simulink model "SdbTxSigProc"  (R2022b) (continued)
== system SdbTxSigProc/SdbTxSigProc_Runnable_sys == (continued)
  HeatrAirTReq_HeatrAirTReq_write : Outport  Port=155
      settings: EnsureOutportIsVirtual=on
  HvacAirMFlowEstimd_HvacAirMFlowEstimd_write : Outport  Port=156
      settings: EnsureOutportIsVirtual=on
  HvacAirMFlowReEstimd_HvacAirMFlowReEstimd_write : Outport  Port=157
      settings: EnsureOutportIsVirtual=on
  HvacAirMFlowVent_HvacAirMFlowVent_write : Outport  Port=158
      settings: EnsureOutportIsVirtual=on
  HvacAirTForRowFirstAtFlrLe_HvacAirTForRowFirstAtFlrLe_write : Outport  Port=159
      settings: EnsureOutportIsVirtual=on
  HvacAirTForRowFirstAtFlrRi_HvacAirTForRowFirstAtFlrRi_write : Outport  Port=160
      settings: EnsureOutportIsVirtual=on
  HvacAirTForRowFirstAtVentLe_HvacAirTForRowFirstAtVentLe_write : Outport  Port=161
      settings: EnsureOutportIsVirtual=on
  HvacAirTForRowFirstAtVentRi_HvacAirTForRowFirstAtVentRi_write : Outport  Port=162
      settings: EnsureOutportIsVirtual=on
  HvacAirTForRowSecAtFlrLe_HvacAirTForRowSecAtFlrLe_write : Outport  Port=163
      settings: EnsureOutportIsVirtual=on
  HvacAirTForRowSecAtFlrRi_HvacAirTForRowSecAtFlrRi_write : Outport  Port=164
      settings: EnsureOutportIsVirtual=on
  HvacAirTForRowSecAtVentLe_HvacAirTForRowSecAtVentLe_write : Outport  Port=165
      settings: EnsureOutportIsVirtual=on
  HvacAirTForRowSecAtVentRi_HvacAirTForRowSecAtVentRi_write : Outport  Port=166
      settings: EnsureOutportIsVirtual=on
  HvacCondsorTsp_HvacCondsorTsp_write : Outport  Port=167
      settings: EnsureOutportIsVirtual=on
  HvacCoolgEnaFrnt_HvacCoolgEnaFrnt_write : Outport  Port=168
      settings: EnsureOutportIsVirtual=on
  HvacCoolgEnaRe_HvacCoolgEnaRe_write : Outport  Port=169
      settings: EnsureOutportIsVirtual=on
  HvacEvaprTSpFrnt_HvacEvaprTSpFrnt_write : Outport  Port=170
      settings: EnsureOutportIsVirtual=on
  HvacHeatElmAirMFlowEstimd_HvacHeatElmAirMFlowEstimd_write : Outport  Port=171
      settings: EnsureOutportIsVirtual=on
  HvacHeatgReq_HvacHeatgReq_write : Outport  Port=172
      settings: EnsureOutportIsVirtual=on
  HvacHeatrInletTempReq_HvacHeatrInletTempReq_write : Outport  Port=173
      settings: EnsureOutportIsVirtual=on
  HvacRecircAct_HvacRecircAct_write : Outport  Port=174
      settings: EnsureOutportIsVirtual=on
  HvEgyDesForClima_HvEgyDesForClima_write : Outport  Port=175
      settings: EnsureOutportIsVirtual=on
  InPm25SnsrActnReq_InPm25SnsrActnReq_write : Outport  Port=176
      settings: EnsureOutportIsVirtual=on
  IntPm25HiPopUp_IntPm25HiPopUp_write : Outport  Port=177
      settings: EnsureOutportIsVirtual=on
  LvlOfClimaCmft_LvlOfClimaCmft_write : Outport  Port=178
      settings: EnsureOutportIsVirtual=on
  PassSeatHeatgPwrAllwd_PassSeatHeatgPwrAllwd_write : Outport  Port=179
      settings: EnsureOutportIsVirtual=on
  PassSeatVentnPwrAllwd_PassSeatVentnPwrAllwd_write : Outport  Port=180
      settings: EnsureOutportIsVirtual=on
  PostDrvgClimaAvl_PostDrvgClimaAvl_write : Outport  Port=181
      settings: EnsureOutportIsVirtual=on
  PwrAllwdForElecAirVentn_PwrAllwdForElecAirVentn_write : Outport  Port=182
      settings: EnsureOutportIsVirtual=on
  PwrEstimdAtStbForClima_PwrEstimdAtStbForClima_write : Outport  Port=183
      settings: EnsureOutportIsVirtual=on
  RlyCmftForClimaReq_RlyCmftForClimaReq_write : Outport  Port=184
      settings: EnsureOutportIsVirtual=on
  RlyCrashForClimaReq_RlyCrashForClimaReq_write : Outport  Port=185
      settings: EnsureOutportIsVirtual=on
  SecRowLeSeatHeatgPwrAllwd_SecRowLeSeatHeatgPwrAllwd_write : Outport  Port=186
      settings: EnsureOutportIsVirtual=on
  SecRowLeSeatVentnPwrAllwd_SecRowLeSeatVentnPwrAllwd_write : Outport  Port=187
      settings: EnsureOutportIsVirtual=on
  SecRowRiSeatHeatgPwrAllwd_SecRowRiSeatHeatgPwrAllwd_write : Outport  Port=188
      settings: EnsureOutportIsVirtual=on
  SecRowRiSeatVentnPwrAllwd_SecRowRiSeatVentnPwrAllwd_write : Outport  Port=189
      settings: EnsureOutportIsVirtual=on
  SteerWhlHeatgDurgClima_SteerWhlHeatgDurgClima_write : Outport  Port=190
      settings: EnsureOutportIsVirtual=on
  TgtHvacAirTForRowFirstLe_TgtHvacAirTForRowFirstLe_write : Outport  Port=191
      settings: EnsureOutportIsVirtual=on
  TgtHvacAirTForRowFirstRi_TgtHvacAirTForRowFirstRi_write : Outport  Port=192
      settings: EnsureOutportIsVirtual=on
  TgtHvacAirTForRowSecLe_TgtHvacAirTForRowSecLe_write : Outport  Port=193
      settings: EnsureOutportIsVirtual=on
  TgtHvacAirTForRowSecRi_TgtHvacAirTForRowSecRi_write : Outport  Port=194
      settings: EnsureOutportIsVirtual=on
  UBoostReqByClima_UBoostReqByClima_write : Outport  Port=195
      settings: EnsureOutportIsVirtual=on
  VehMktForPmSnsr_VehMktForPmSnsr_write : Outport  Port=196
      settings: EnsureOutportIsVirtual=on
  WindIceDetdFrnt_WindIceDetdFrnt_write : Outport  Port=197
      settings: EnsureOutportIsVirtual=on
  WindIceDetdRe_WindIceDetdRe_write : Outport  Port=198
      settings: EnsureOutportIsVirtual=on
  wires:
  AirTAftHvacDes_AirTAftHvacDes_read/1 -> Terminator_1/1
  AirTFbFromClima_Ngcc_AirTFbFromClima_read/1 -> Terminator_2/1
  AmbTEstimd_Ngcc_AmbTEstimd_read/1 -> Terminator_3/1
  AmbTFildForClimaNgcc_AmbTFildForClima_read/1 -> Terminator_4/1
  AmbTFildForClimaQf_AmbTFildForClimaQf_read/1 -> Terminator_5/1
  CdsnProbltyToExt_CdsnProbltyToExt_read/1 -> Terminator_6/1
  ClimaActv_Ngcc_ClimaActv_read/1 -> Terminator_7/1
  ClimaActvAlrmSts_Ngcc_ClimaActvAlrmSts_read/1 -> Terminator_8/1
  ClimaCoolgPwrThermPred_ClimaCoolgPwrThermPred_read/1 -> Terminator_9/1
  ClimaDefrstMaxReq_ClimaDefrstMaxReq_read/1 -> Terminator_10/1
  ClimaOffReq_Ngcc_ClimaOffReq_read/1 -> Terminator_11/1
  ClimaPwrCns_Ngcc_ClimaPwrCns_read/1 -> Terminator_12/1
  ClimaPwrCnsPred_ClimaPwrCnsPred_read/1 -> Terminator_13/1
  ClimaPwrCritDes_Ngcc_ClimaPwrCritDes_read/1 -> Terminator_14/1
  ClimaPwrNormDes_Ngcc_ClimaPwrNormDes_read/1 -> Terminator_15/1
  ClimaStrtTi_ClimaStrtTi_read/1 -> Terminator_16/1
  ClimaSts_Ngcc_ClimaSts_read/1 -> Terminator_17/1
  ClimaTiOfEnd_Ngcc_ClimaTiOfEnd_read/1 -> Terminator_18/1
  ClimaTmrSts_ClimaTmrSts_read/1 -> Terminator_19/1
  ClimaWarn_ClimaWarn_read/1 -> Terminator_20/1
  ClngActv_ClngActv_read/1 -> Terminator_21/1
  CmptmtAirTEstimdAtRowFirstLoResl_Ngcc_CmptmtAirTEstimdAtRowFirstLoResl_read/1 -> Terminator_22/1
  CmptmtAirTEstimdExtd_Ngcc_CmptmtAirTEstimdExtd_read/1 -> Terminator_23/1
  CmptmtAirTFbExtd_CmptmtAirTFbExtd_read/1 -> Terminator_24/1
  CmptmtRelHumToExt_CmptmtRelHumToExt_read/1 -> Terminator_25/1
  CoolgStsForDisp_CoolgStsForDisp_read/1 -> Terminator_26/1
  CupHoldrActvAllwd_Ngcc_CupHoldrActvAllwd_read/1 -> Terminator_27/1
  CustClimaOvrdNotifForHmiCpt_CustClimaOvrdNotifForHmiCpt_read/1 -> Terminator_28/1
  DefrstReq_DefrstReq_read/1 -> Terminator_29/1
  DewVal_DewVal_read/1 -> Terminator_30/1
  DrvrSeatHeatgPwrAllwd_Ngcc_DrvrSeatHeatgPwrAllwd_read/1 -> Terminator_31/1
  DrvrSeatVentnPwrAllwd_Ngcc_DrvrSeatVentnPwrAllwd_read/1 -> Terminator_32/1
  EcoClimasts_Ngcc_EcoClimasts_read/1 -> Terminator_33/1
  EcoModForClimaActv_EcoModForClimaActv_read/1 -> Terminator_34/1
  EgyDesForClima_Ngcc_EgyDesForClima_read/1 -> Terminator_35/1
  EngRunngReqByClima_Ngcc_EngRunngReqByClima_read/1 -> Terminator_36/1
  EngSpdIncIdleForClima_Ngcc_EngSpdIncIdleForClima_read/1 -> Terminator_37/1
  EvaprTFrntFildForClima_EvaprTFrntFildForClima_read/1 -> Terminator_38/1
  EvaprTFrntFildQfForClima_EvaprTFrntFildQfForClima_read/1 -> Terminator_39/1
  HmiDefrstReqForClima_HmiDefrstReqForClima_read/1 -> Terminator_40/1
  HvacAirMFlowEstimd_Ngcc_HvacAirMFlowEstimd_read/1 -> Terminator_41/1
  HvacAirMFlowEstimdInHeatElm_HvacAirMFlowEstimdInHeatElm_read/1 -> Terminator_42/1
  HvacAirMFlowReEstimd_Ngcc_HvacAirMFlowReEstimd_read/1 -> Terminator_43/1
  HvacAirTFildForRowFirstAtFlrLe_HvacAirTFildForRowFirstAtFlrLe_read/1 -> Terminator_44/1
  HvacAirTFildForRowFirstAtFlrRi_HvacAirTFildForRowFirstAtFlrRi_read/1 -> Terminator_45/1
  HvacAirTFildForRowFirstAtVentLe_HvacAirTFildForRowFirstAtVentLe_read/1 -> Terminator_46/1
  HvacAirTFildForRowFirstAtVentRi_HvacAirTFildForRowFirstAtVentRi_read/1 -> Terminator_47/1
  HvacAirTFildForRowSecAtFlrLe_HvacAirTFildForRowSecAtFlrLe_read/1 -> Terminator_48/1
  HvacAirTFildForRowSecAtFlrRi_HvacAirTFildForRowSecAtFlrRi_read/1 -> Terminator_49/1
  HvacAirTFildForRowSecAtVentLe_HvacAirTFildForRowSecAtVentLe_read/1 -> Terminator_50/1
  HvacAirTFildForRowSecAtVentRi_HvacAirTFildForRowSecAtVentRi_read/1 -> Terminator_51/1
  HvacAirTForRowFirstAtFlrLeRaw_HvacAirTForRowFirstAtFlrLeRaw_read/1 -> Terminator_52/1
  HvacAirTForRowFirstAtFlrRiRaw_HvacAirTForRowFirstAtFlrRiRaw_read/1 -> Terminator_53/1
  HvacAirTForRowFirstAtVentRiRaw_HvacAirTForRowFirstAtVentRiRaw_read/1 -> Terminator_54/1
  HvacAirTForRowSecAtFlrLeRaw_HvacAirTForRowSecAtFlrLeRaw_read/1 -> Terminator_55/1
  HvacAirTForRowSecAtFlrRiRaw_HvacAirTForRowSecAtFlrRiRaw_read/1 -> Terminator_56/1
  HvacAirTForRowSecAtVentLeRaw_HvacAirTForRowSecAtVentLeRaw_read/1 -> Terminator_57/1
  HvacAirTForRowSecAtVentRiRaw_HvacAirTForRowSecAtVentRiRaw_read/1 -> Terminator_58/1
  HvacAirTReqAtRowFirstLe_HvacAirTReqAtRowFirstLe_read/1 -> Terminator_59/1
  HvacAirTReqAtRowFirstRi_HvacAirTReqAtRowFirstRi_read/1 -> Terminator_60/1
  HvacAirTReqAtRowSecLe_HvacAirTReqAtRowSecLe_read/1 -> Terminator_61/1
  HvacAirTReqAtRowSecRi_HvacAirTReqAtRowSecRi_read/1 -> Terminator_62/1
  HvacCondsorTspEvaprTFrnt_HvacCondsorTspEvaprTFrnt_read/1 -> Terminator_63/1
  HvacCoolgEnaFrnt_Ngcc_HvacCoolgEnaFrnt_read/1 -> Terminator_64/1
  HvacCoolgEnaRe_Ngcc_HvacCoolgEnaRe_read/1 -> Terminator_65/1
  HvacEvaprTSpFrnt_Ngcc_HvacEvaprTSpFrnt_read/1 -> Terminator_66/1
  HvacFlowFrntActForRowFirstAtVent_HvacFlowFrntActForRowFirstAtVent_read/1 -> Terminator_67/1
  HvacFlowFrntActForRowFirstAtVent_HvacFlowFrntActForRowFirstAtVentLe_read/1 -> Terminator_68/1
  HvacFlowFrntActForRowFirstAtVent_HvacFlowFrntActForRowFirstAtVentRi_read/1 -> Terminator_69/1
  HvacFlowFrntActForRowFirstAtVent_HvacFlowFrntActForRowFirstAtVentSideLe_read/1 -> Terminator_70/1
  HvacFlowFrntActForRowFirstAtVent_HvacFlowFrntActForRowFirstAtVentSideRi_read/1 -> Terminator_71/1
  HvacHeatgReqInt_HvacHeatgReqInt_read/1 -> Terminator_72/1
  HvacRecircAct_Ngcc_HvacRecircAct_read/1 -> Terminator_73/1
  HvEgyDesForClima_Ngcc_HvEgyDesForClima_read/1 -> Terminator_74/1
  InPm25SnsrActnReq_Ngcc_InPm25SnsrActnReq_read/1 -> Terminator_75/1
  IntPm25HiPopUp_Ngcc_IntPm25HiPopUp_read/1 -> Terminator_76/1
  LvlOfClimaCmft_Ngcc_LvlOfClimaCmft_read/1 -> Terminator_77/1
  PassSeatHeatgPwrAllwd_Ngcc_PassSeatHeatgPwrAllwd_read/1 -> Terminator_78/1
  PassSeatVentnPwrAllwd_Ngcc_PassSeatVentnPwrAllwd_read/1 -> Terminator_79/1
  PostDrvgClimaAvl_Ngcc_PostDrvgClimaAvl_read/1 -> Terminator_80/1
  PreClngActv_PreClngActv_read/1 -> Terminator_81/1
  PreClngNotif_PreClngNotif_read/1 -> Terminator_82/1
  PwrAllwdForElecAirVentn_Ngcc_PwrAllwdForElecAirVentn_read/1 -> Terminator_83/1
  PwrAllwdForSeatHeatg_PwrAllwdForSeatHeatg_read/1 -> Terminator_84/1
  PwrAllwdForSeatVent_PwrAllwdForSeatVent_read/1 -> Terminator_85/1
  PwrChrgDetdForPrkgHeatrElec_PwrChrgDetdForPrkgHeatrElec_read/1 -> Terminator_86/1
  PwrCnsHeatgElecPred_PwrCnsHeatgElecPred_read/1 -> Terminator_87/1
  PwrEstimdAtStbForClima_Ngcc_PwrEstimdAtStbForClima_read/1 -> Terminator_88/1
  RlyCmftForClimaReq_Ngcc_RlyCmftForClimaReq_read/1 -> Terminator_89/1
  RlyCrashForClimaReq_Ngcc_RlyCrashForClimaReq_read/1 -> Terminator_90/1
  SeatHeatgAutCdn_SeatHeatgAutCdn_read/1 -> Terminator_91/1
  SecRowLeSeatHeatgPwrAllwd_Ngcc_SecRowLeSeatHeatgPwrAllwd_read/1 -> Terminator_92/1
  SecRowLeSeatVentnPwrAllwd_Ngcc_SecRowLeSeatVentnPwrAllwd_read/1 -> Terminator_93/1
  SecRowRiSeatHeatgPwrAllwd_Ngcc_SecRowRiSeatHeatgPwrAllwd_read/1 -> Terminator_94/1
  SecRowRiSeatVentnPwrAllwd_Ngcc_SecRowRiSeatVentnPwrAllwd_read/1 -> Terminator_95/1
  SteerWhlHeatgAllwd_SteerWhlHeatgAllwd_read/1 -> Terminator_96/1
  SteerWhlHeatgDurgClima_Ngcc_SteerWhlHeatgDurgClima_read/1 -> Terminator_97/1
  UBoostReqByClima_Ngcc_UBoostReqByClima_read/1 -> Terminator_98/1
  VehMktForPmSnsr_Ngcc_VehMktForPmSnsr_read/1 -> Terminator_99/1
  WindDewUppr_WindDewTUppr_read/1 -> Terminator_100/1
  WindIceDetdFrnt_Ngcc_WindIceDetdFrnt_read/1 -> Terminator_101/1
  WindIceDetdRe_Ngcc_WindIceDetdRe_read/1 -> Terminator_102/1
  WindT_WindT_read/1 -> Terminator_103/1
  HvacAirTForRowFirstAtVentLeRaw_HvacAirTForRowFirstAtVentLeRaw_read/1 -> Terminator_104/1
  ClimaStStrtUp_ClimaStStrtUp_read/1 -> Terminator_105/1
  AACAirClngActnNgcc_AACAirClngActnNgcc_read/1 -> Terminator_106/1
  AirSrcReqNgcc_AirSrcReqNgcc_read/1 -> Terminator_107/1
  AirTAftHvacDesFirstLe_AirTAftHvacDesFirstLe_read/1 -> Terminator_108/1
  AirTAftHvacDesFirstRi_AirTAftHvacDesFirstRi_read/1 -> Terminator_109/1
  AirTAftHvacDesRe_AirTAftHvacDesRe_read/1 -> Terminator_110/1
  AirTAftHvacDesSecLe_AirTAftHvacDesSecLe_read/1 -> Terminator_111/1
  AirTFromHeatgEstimdFirstLeForCCM_AirTFromHeatgEstimdFirstLeForCCM_read/1 -> Terminator_112/1
  AirTFromHeatgEstimdFirstRiForCCM_AirTFromHeatgEstimdFirstRiForCCM_read/1 -> Terminator_113/1
  AirTFromHeatgEstimdForCCM_AirTFromHeatgEstimd_read/1 -> Terminator_114/1
  AirTFromHeatgEstimdReForCCM_AirTFromHeatgEstimdReForCCM_read/1 -> Terminator_115/1
  AirTFromHeatgEstimdSecLeForCCM_AirTFromHeatgEstimdSecLeForCCM_read/1 -> Terminator_116/1
  AutOxygenSplyStsFrmClimaNgcc_AutOxygenSplyStsFrmClimaNgcc_read/1 -> Terminator_117/1
  CcmAACAirClngStsNgcc_CcmAACAirClngStsNgcc_read/1 -> Terminator_118/1
  CCMDataTraceZCUP1Ngcc_CCMDataTraceZCUP1Ngcc_read/1 -> Terminator_119/1
  CCMDataTraceZCUP2Ngcc_CCMDataTraceZCUP2Ngcc_read/1 -> Terminator_120/1
  CCMDataTraceZCUP3Ngcc_CCMDataTraceZCUP3Ngcc_read/1 -> Terminator_121/1
  CCMDataTraceZCUP4Ngcc_CCMDataTraceZCUP4Ngcc_read/1 -> Terminator_122/1
  CCMDataTraceZCUP5Ngcc_CCMDataTraceZCUP5Ngcc_read/1 -> Terminator_123/1
  CCMDataTraceZCUP6Ngcc_CCMDataTraceZCUP6Ngcc_read/1 -> Terminator_124/1
  CCMDataTraceZCUP7Ngcc_CCMDataTraceZCUP7Ngcc_read/1 -> Terminator_125/1
  CcmElecAirDirCrtlReqSecCnsLePosXNgcc_CcmElecAirDirCrtlReqSecCnsLePosXNgcc_read/1 -> Terminator_126/1
  CcmElecAirDirCrtlReqSecCnsLePosYNgcc_CcmElecAirDirCrtlReqSecCnsLePosYNgcc_read/1 -> Terminator_127/1
  CcmElecAirDirCrtlReqSecCnsRiPosXNgcc_CcmElecAirDirCrtlReqSecCnsRiPosXNgcc_read/1 -> Terminator_128/1
  CcmElecAirDirCrtlReqSecCnsRiPosYNgcc_CcmElecAirDirCrtlReqSecCnsRiPosYNgcc_read/1 -> Terminator_129/1
  CCMFragCh1OvrdDyNgcc_CCMFragCh1OvrdDyNgcc_read/1 -> Terminator_130/1
  CCMFragCh2OvrdDyNgcc_CCMFragCh2OvrdDyNgcc_read/1 -> Terminator_131/1
  CCMFragCh3OvrdDyNgcc_CCMFragCh3OvrdDyNgcc_read/1 -> Terminator_132/1
  CCMFragCh4OvrdDyNgcc_CCMFragCh4OvrdDyNgcc_read/1 -> Terminator_133/1
  CCMFragCh5OvrdDyNgcc_CCMFragCh5OvrdDyNgcc_read/1 -> Terminator_134/1
  CCMFragraCh1AvlTiPrecNgcc_CCMFragraCh1AvlTiPrecNgcc_read/1 -> Terminator_135/1
  CCMFragraCh1AvlTiPrecNgcc_CCMFragraCh1AvlTiPrecNgcc_IsUpdated_read/1 -> Terminator_136/1
  CCMFragraCh2AvlTiPrecNgcc_CCMFragraCh2AvlTiPrecNgcc_read/1 -> Terminator_137/1
  CCMFragraCh2AvlTiPrecNgcc_CCMFragraCh2AvlTiPrecNgcc_IsUpdated_read/1 -> Terminator_138/1
  CCMFragraCh3AvlTiPrecNgcc_CCMFragraCh3AvlTiPrecNgcc_read/1 -> Terminator_139/1
  CCMFragraCh3AvlTiPrecNgcc_CCMFragraCh3AvlTiPrecNgcc_IsUpdated_read/1 -> Terminator_140/1
  CCMFragraCh4AvlTiPrecNgcc_CCMFragraCh4AvlTiPrecNgcc_read/1 -> Terminator_141/1
  CCMFragraCh4AvlTiPrecNgcc_CCMFragraCh4AvlTiPrecNgcc_IsUpdated_read/1 -> Terminator_142/1
  CCMFragraCh5AvlTiPrecNgcc_CCMFragraCh5AvlTiPrecNgcc_read/1 -> Terminator_143/1
  CCMFragraCh5AvlTiPrecNgcc_CCMFragraCh5AvlTiPrecNgcc_IsUpdated_read/1 -> Terminator_144/1
  CcmWbmMasterReqLeLin1Ngcc_CcmWbmMasterReqLeLin1Ngcc_read/1 -> Terminator_145/1
  CcmWbmMasterReqRiLin1Ngcc_CcmWbmMasterReqRiLin1Ngcc_read/1 -> Terminator_146/1
  CdsnProblty_CdsnProblty_read/1 -> Terminator_147/1
  ChgFilElmRstNgcc_ChgFilElmRstNgcc_read/1 -> Terminator_148/1
  ClimaAtRowSecActvNgcc_ClimaAtRowSecActv_read/1 -> Terminator_149/1
  ClimaAtRowSecActvNgcc_ClimaAtRowSecActvLe_read/1 -> Terminator_150/1
  ClimaAtRowSecActvNgcc_ClimaAtRowSecActvRi_read/1 -> Terminator_151/1
  ClimaCtrlActvNgcc_ClimaCtrlActv_read/1 -> Terminator_152/1
  ClimaManModCmftPopUpReqNgcc_ClimaManModCmftPopUpReqNgcc_read/1 -> Terminator_153/1
  CmptmtAirDistbnRspNgcc_CmptmtAirDistbnRspNgcc_read/1 -> Terminator_154/1
  CmptmtAirDistbnRspNgcc_CmptmtAirDistbnRspNgcc_IsUpdated_read/1 -> Terminator_155/1
  CmptmtOxygenConcLvlFrmClimaNgcc_CmptmtOxygenConcLvlFrmClimaNgcc_read/1 -> Terminator_156/1
  CmptmtOxygenConcVluFrmClimaNgcc_CmptmtOxygenConcVluFrmClimaNgcc_read/1 -> Terminator_157/1
  CoupldCtrlErrAtRowFirstLeNgcc_CoupldCtrlErrAtRowFirstLe_read/1 -> Terminator_158/1
  CoupldCtrlErrAtRowFirstRiNgcc_CoupldCtrlErrAtRowFirstRi_read/1 -> Terminator_159/1
  CoupldCtrlErrAtRowSecLeNgcc_CoupldCtrlErrAtRowSecLe_read/1 -> Terminator_160/1
  CoupldCtrlErrAtRowSecRiNgcc_CoupldCtrlErrAtRowSecRi_read/1 -> Terminator_161/1
  DutyCmdForMosBlw_DutyCmdForMosBlw_read/1 -> Terminator_162/1
  DutyCmdForPWMBlw_DutyCmdForPWMBlw_read/1 -> Terminator_163/1
  ExtVentToastFrmClima_Ngcc_ExtVentToastFrmClima_read/1 -> Terminator_164/1
  FrntClimaManModCmftStsNgcc_FrntClimaManModCmftStsNgcc_read/1 -> Terminator_165/1
  HighTempCleanRunningStsNgcc_HighTempCleanRunningStsNgcc_read/1 -> Terminator_166/1
  HighTempCleanSettingStsNgcc_HighTempCleanSettingStsNgcc_read/1 -> Terminator_167/1
  HvacAirTReAtFlrLeRaw_HvacAirTReAtFlrLeRaw_read/1 -> Terminator_168/1
  HvacAirTReAtVentLeRaw_HvacAirTReAtVentLeRaw_read/1 -> Terminator_169/1
  HvacEvaprTSpReNgcc_HvacEvaprTSpReNgcc_read/1 -> Terminator_170/1
  HvacFanLvlFbNgcc_HvacFanLvlFbNgcc_read/1 -> Terminator_171/1
  HvacFanLvlFbReNgcc_HvacFanLvlFbReNgcc_read/1 -> Terminator_172/1
  HvacHeatElmAirMFlowEstimdFirstLeNgcc_HvacHeatElmAirMFlowEstimdFirstLeNgcc_read/1 -> Terminator_173/1
  HvacHeatElmAirMFlowEstimdFirstRiNgcc_HvacHeatElmAirMFlowEstimdFirstRiNgcc_read/1 -> Terminator_174/1
  HvacHeatElmAirMFlowEstimdSecLeNgcc_HvacHeatElmAirMFlowEstimdSecLeNgcc_read/1 -> Terminator_175/1
  HvacHeatgReqReInt_HvacHeatgReqReInt_read/1 -> Terminator_176/1
  HvacHexAirTForRowFirstLeRaw_HvacHexAirTForRowFirstLeRaw_read/1 -> Terminator_177/1
  HvacHexAirTForRowFirstRiRaw_HvacHexAirTForRowFirstRiRaw_read/1 -> Terminator_178/1
  HvacHexAirTForRowSecLeRaw_HvacHexAirTForRowSecLeRaw_read/1 -> Terminator_179/1
  KeySynchrnztnLclNgcc_KeySynchrnztnLclNgcc_read/1 -> Terminator_180/1
  OutlSovActvStsNgcc_OutlSovActvStsNgcc_read/1 -> Terminator_181/1
  OxygenConcSnsrActnReqNgcc_OxygenConcSnsrActnReqNgcc_read/1 -> Terminator_182/1
  OxygenConcSnsrStsFrmClimaNgcc_OxygenConcSnsrStsFrmClimaNgcc_read/1 -> Terminator_183/1
  OxygenSplyAvlStsFrmClimaNgcc_OxygenSplyAvlStsFrmClimaNgcc_read/1 -> Terminator_184/1
  OxygenSplyErrFrmClimaNgcc_OxygenSplyErrFrmClimaNgcc_read/1 -> Terminator_185/1
  OxygenSplyPopUpReqNgcc_OxygenSplyPopUpReqNgcc_read/1 -> Terminator_186/1
  OxygenSplyPwrAllwdNgcc_OxygenSplyPwrAllwdNgcc_read/1 -> Terminator_187/1
  OxygenSplyReqFaildNgcc_OxygenSplyReqFaildNgcc_read/1 -> Terminator_188/1
  OxygenSplyReqNgcc_OxygenSplyReqNgcc_read/1 -> Terminator_189/1
  OxygenSplyVoiceFbNgcc_OxygenSplyVoiceFbNgcc_read/1 -> Terminator_190/1
  PrkgClimaEngStReqNgcc_PrkgClimaEngStReqNgcc_read/1 -> Terminator_191/1
  PwrCnsForHvacFanFrntNgcc_PwrCnsForHvacFanFrnt_read/1 -> Terminator_192/1
  PwrCnsForHvacFanReNgcc_PwrCnsForHvacFanRe_read/1 -> Terminator_193/1
  ReClimaManModCmftStsNgcc_ReClimaManModCmftStsNgcc_read/1 -> Terminator_194/1
  RemOxygenSplyActvFaildNgcc_RemOxygenSplyActvFaildNgcc_read/1 -> Terminator_195/1
  TCSCmprStrtFltNgcc_TCSCmprStrtFltNgcc_read/1 -> Terminator_196/1
  TCScoolgGrdReqNgcc_TCScoolgGrdReqNgcc_read/1 -> Terminator_197/1
  TCSCooltFlowReq1Ngcc_TCSCooltFlowReq1Ngcc_read/1 -> Terminator_198/1
  TCSCooltTReq1Ngcc_TCSCooltTReq1Ngcc_read/1 -> Terminator_199/1
  TCSDoorStsNgcc_TCSDoorStsNgcc_read/1 -> Terminator_200/1
  TCSDoorTopSts1Ngcc_TCSDoorTopSts1Ngcc_read/1 -> Terminator_201/1
  TcsEcoModSts1Ngcc_TcsEcoModSts1Ngcc_read/1 -> Terminator_202/1
  TcsFltNewSts1Ngcc_TcsFltNewSts1Ngcc_read/1 -> Terminator_203/1
  TCSFltStsNgcc_TCSFltStsNgcc_read/1 -> Terminator_204/1
  TCSModSwtStsNgcc_TCSModSwtStsNgcc_read/1 -> Terminator_205/1
  TCSnsrTStsNgcc_TCSnsrTStsNgcc_read/1 -> Terminator_206/1
  TCSRadrTreqNgcc_TCSRadrTreqNgcc_read/1 -> Terminator_207/1
  TCSRadrTStsNgcc_TCSRadrTStsNgcc_read/1 -> Terminator_208/1
  TCSTmrOverStsNgcc_TCSTmrOverStsNgcc_read/1 -> Terminator_209/1
  TCSTmrStrtStsNgcc_TCSTmrStrtStsNgcc_read/1 -> Terminator_210/1
  TCSTNowStsNgcc_TCSTNowStsNgcc_read/1 -> Terminator_211/1
  TgtHvacAirTForMaxTZoneNgcc_TgtHvacAirTForMaxTZoneNgcc_read/1 -> Terminator_212/1
  TgtHvacAirTForRowFirstLeNgcc_TgtHvacAirTForRowFirstLeNgcc_read/1 -> Terminator_213/1
  TgtHvacAirTForRowFirstRiNgcc_TgtHvacAirTForRowFirstRiNgcc_read/1 -> Terminator_214/1
  TgtHvacAirTForRowSecLeNgcc_TgtHvacAirTForRowSecLeNgcc_read/1 -> Terminator_215/1
  TgtHvacAirTForRowSecRiNgcc_TgtHvacAirTForRowSecRiNgcc_read/1 -> Terminator_216/1
  ThermoTSteDeoSts1Ngcc_ThermoTSteDeoSts1Ngcc_read/1 -> Terminator_217/1
  ThrdRowLeSeatHeatgPwrAllwdNgcc_ThrdRowLeSeatHeatgPwrAllwdNgcc_read/1 -> Terminator_218/1
  ThrdRowLeSeatVentnPwrAllwdNgcc_ThrdRowLeSeatVentnPwrAllwdNgcc_read/1 -> Terminator_219/1
  ThrdRowRiSeatHeatgPwrAllwdNgcc_ThrdRowRiSeatHeatgPwrAllwdNgcc_read/1 -> Terminator_220/1
  ThrdRowRiSeatVentnPwrAllwdNgcc_ThrdRowRiSeatVentnPwrAllwdNgcc_read/1 -> Terminator_221/1
  TiOfDeactvnForClimaCtrlNgcc_TiOfDeactvnForClimaCtrl_read/1 -> Terminator_222/1
  VMMChngNdMtnNgcc_VMMChngNdMtnNgcc_read/1 -> Terminator_223/1
  Ground_1/1 -> AACAirClngActn_AACAirClngActn_write/1
  Ground_2/1 -> AirSrcReq_AirSrcReq_write/1
  Ground_3/1 -> AmbTFildForClima_AmbTFildForClima_write/1
  Ground_4/1 -> AutOxygenSplyStsFrmClima_AutOxygenSplyStsFrmClima_write/1
  Ground_5/1 -> CcmAACAirClngSts_CcmAACAirClngSts_write/1
  Ground_6/1(Out1) -> CCMDataTraceZCUP1_CCMDataTraceZCUP1_write/1
  Ground_7/1(Out1) -> CCMDataTraceZCUP2_CCMDataTraceZCUP2_write/1
  Ground_8/1(Out1) -> CCMDataTraceZCUP3_CCMDataTraceZCUP3_write/1
  Ground_9/1(Out1) -> CCMDataTraceZCUP4_CCMDataTraceZCUP4_write/1
  Ground_10/1(Out1) -> CCMDataTraceZCUP5_CCMDataTraceZCUP5_write/1
  Ground_11/1(Out1) -> CCMDataTraceZCUP6_CCMDataTraceZCUP6_write/1
  Ground_12/1(Out1) -> CCMDataTraceZCUP7_CCMDataTraceZCUP7_write/1
  Ground_13/1 -> CcmElecAirDirCrtlReqSecCnsLePosX_CcmElecAirDirCrtlReqSecCnsLePosX_write/1
  Ground_14/1 -> CcmElecAirDirCrtlReqSecCnsLePosY_CcmElecAirDirCrtlReqSecCnsLePosY_write/1
  Ground_15/1 -> CcmElecAirDirCrtlReqSecCnsRiPosX_CcmElecAirDirCrtlReqSecCnsRiPosX_write/1
  Ground_16/1 -> CcmElecAirDirCrtlReqSecCnsRiPosY_CcmElecAirDirCrtlReqSecCnsRiPosY_write/1
  Ground_17/1 -> CCMFragCh1OvrdDy_CCMFragCh1OvrdDy_write/1
  Ground_18/1 -> CCMFragCh2OvrdDy_CCMFragCh2OvrdDy_write/1
  Ground_19/1 -> CCMFragCh3OvrdDy_CCMFragCh3OvrdDy_write/1
  Ground_20/1 -> CCMFragCh4OvrdDy_CCMFragCh4OvrdDy_write/1
  Ground_21/1 -> CCMFragCh5OvrdDy_CCMFragCh5OvrdDy_write/1
  Ground_22/1 -> CCMFragraCh1AvlTiPrec_CCMFragraCh1AvlTiPrec_write/1
  Ground_23/1 -> CCMFragraCh2AvlTiPrec_CCMFragraCh2AvlTiPrec_write/1
  Ground_24/1 -> CCMFragraCh3AvlTiPrec_CCMFragraCh3AvlTiPrec_write/1
  Ground_25/1 -> CCMFragraCh4AvlTiPrec_CCMFragraCh4AvlTiPrec_write/1
  Ground_26/1 -> CCMFragraCh5AvlTiPrec_CCMFragraCh5AvlTiPrec_write/1
  Ground_27/1 -> CcmWbmMasterReqLeLin1_CcmWbmMasterReqLeLin1_write/1
  Ground_28/1 -> CcmWbmMasterReqRiLin1_CcmWbmMasterReqRiLin1_write/1
  Ground_29/1 -> ChgFilElmRst_ChgFilElmRst_write/1
  Ground_30/1 -> ClimaAtRowSecActv_ClimaAtRowSecActv_write/1
  Ground_31/1 -> ClimaCtrlActv_ClimaCtrlActv_write/1
  Ground_32/1 -> ClimaCtrlTiOfDeactvn_ClimaCtrlTiOfDeactvn_write/1
  Ground_33/1 -> ClimaEstimdTForHeat_ClimaEstimdTForHeat_write/1
  Ground_34/1 -> ClimaEstimdTForReHeat_ClimaEstimdTForReHeat_write/1
  Ground_35/1 -> ClimaManModCmftPopUpReq_ClimaManModCmftPopUpReq_write/1
  Ground_36/1(Out1) -> CmptmtAirDistbnRsp_CmptmtAirDistbnRsp_write/1
  Ground_37/1 -> CmptmtOxygenConcLvlFrmClima_CmptmtOxygenConcLvlFrmClima_write/1
  Ground_38/1 -> CmptmtOxygenConcVluFrmClima_CmptmtOxygenConcVluFrmClima_write/1
  Ground_39/1 -> CoupldCtrlErrAtRowFirstLe_CoupldCtrlErrAtRowFirstLe_write/1
  Ground_40/1 -> CoupldCtrlErrAtRowFirstRi_CoupldCtrlErrAtRowFirstRi_write/1
  Ground_41/1 -> CoupldCtrlErrAtRowSecLe_CoupldCtrlErrAtRowSecLe_write/1
  Ground_42/1 -> CoupldCtrlErrAtRowSecRi_CoupldCtrlErrAtRowSecRi_write/1
  Ground_43/1 -> FrntBlwSpdReq_FrntBlwSpdReq_write/1
  Ground_44/1 -> FrntClimaManModCmftSts_FrntClimaManModCmftSts_write/1
  Ground_45/1 -> HeatrAirTReqFirstLe_HeatrAirTReqFirstLe_write/1
  Ground_46/1 -> HeatrAirTReqFirstRi_HeatrAirTReqFirstRi_write/1
  Ground_47/1 -> HeatrAirTReqSecLe_HeatrAirTReqSecLe_write/1
  Ground_48/1 -> HighTempCleanRunningSts_HighTempCleanRunningSts_write/1
  Ground_49/1 -> HighTempCleanSettingSts_HighTempCleanSettingSts_write/1
  Ground_50/1(Out1) -> HvacAirTForRowThrdAtFlrRi_HvacAirTForRowThrdAtFlrRi_write/1
  Ground_51/1(Out1) -> HvacAirTForRowThrdAtVentRi_HvacAirTForRowThrdAtVentRi_write/1
  Ground_52/1 -> HvacEvaprTSpRe_HvacEvaprTSpRe_write/1
  Ground_53/1 -> HvacFanLvlFbFrnt_HvacFanLvlFbFrnt_write/1
  Ground_54/1 -> HvacFanLvlFbRe_HvacFanLvlFbRe_write/1
  Ground_55/1 -> HvacHeatElmAirMFlowEstimdFirstLe_HvacHeatElmAirMFlowEstimdFirstLe_write/1
  Ground_56/1 -> HvacHeatElmAirMFlowEstimdFirstRi_HvacHeatElmAirMFlowEstimdFirstRi_write/1
  Ground_57/1 -> HvacHeatElmAirMFlowEstimdSecLe_HvacHeatElmAirMFlowEstimdSecLe_write/1
  Ground_58/1(Out1) -> HvacHexAirTFirstLe_HvacHexAirTFirstLe_write/1
  Ground_59/1(Out1) -> HvacHexAirTFirstRi_HvacHexAirTFirstRi_write/1
  Ground_60/1(Out1) -> HvacHexAirTSecLe_HvacHexAirTSecLe_write/1
  Ground_61/1 -> HvahAirTsp_HvahAirTsp_write/1
  Ground_62/1 -> HvahHeatgReq_HvahHeatgReq_write/1
  Ground_63/1 -> HwFrntBlwPwmReq_HwFrntBlwPwmReq_write/1
  Ground_64/1(Out1) -> KeySynchrnztnLcl_KeySynchrnztnLcl_write/1
  Ground_65/1 -> MistRiskLvl_MistRiskLvl_write/1
  Ground_66/1 -> OutlSovActvSts_OutlSovActvSts_write/1
  Ground_67/1 -> OxygenConcSnsrActnReq_OxygenConcSnsrActnReq_write/1
  Ground_68/1 -> OxygenConcSnsrStsFrmClima_OxygenConcSnsrStsFrmClima_write/1
  Ground_69/1 -> OxygenSplyAvlStsFrmClima_OxygenSplyAvlStsFrmClima_write/1
  Ground_70/1 -> OxygenSplyErrFrmClima_OxygenSplyErrFrmClima_write/1
  Ground_71/1 -> OxygenSplyPopUpReq_OxygenSplyPopUpReq_write/1
  Ground_72/1 -> OxygenSplyPwrAllwd_OxygenSplyPwrAllwd_write/1
  Ground_73/1 -> OxygenSplyReq_OxygenSplyReq_write/1
  Ground_74/1 -> OxygenSplyReqFaild_OxygenSplyReqFaild_write/1
  Ground_75/1 -> OxygenSplyVoiceFb_OxygenSplyVoiceFb_write/1
  Ground_76/1 -> PrkgClimaEngStReq_PrkgClimaEngStReq_write/1
  Ground_77/1 -> PwrCnsForHvacFanFrnt_PwrCnsForHvacFanFrnt_write/1
  Ground_78/1 -> PwrCnsForHvacFanRe_PwrCnsForHvacFanRe_write/1
  Ground_79/1 -> ReClimaManModCmftSts_ReClimaManModCmftSts_write/1
  Ground_80/1(Out1) -> RemClimaElecDefrstReq_RemClimaElecDefrstReq_write/1
  Ground_81/1 -> RemOxygenSplyActvFaild_RemOxygenSplyActvFaild_write/1
  Ground_82/1 -> TCSCmprStrtFlt_TCSCmprStrtFlt_write/1
  Ground_83/1 -> TCSCmprStrtFlt1_TCSCmprStrtFlt1_write/1
  Ground_84/1 -> TCScoolgGrdReq_TCScoolgGrdReq_write/1
  Ground_85/1 -> TCScoolgGrdReq1_TCScoolgGrdReq1_write/1
  Ground_86/1 -> TCSCooltFlowReq1_TCSCooltFlowReq1_write/1
  Ground_87/1 -> TCSCooltTReq1_TCSCooltTReq1_write/1
  Ground_88/1 -> TCSDoorSts_TCSDoorSts_write/1
  Ground_89/1 -> TCSDoorSts1_TCSDoorSts1_write/1
  Ground_90/1 -> TCSDoorTopSts1_TCSDoorTopSts1_write/1
  Ground_91/1 -> TcsEcoModSts1_Element_write/1
  Ground_92/1(Out1) -> TcsFltNewSts1_TcsFltNewSts1_write/1
  Ground_93/1 -> TCSFltSts_TCSFltSts_write/1
  Ground_94/1 -> TCSFltSts1_TCSFltSts1_write/1
  Ground_95/1 -> TCSModSwtSts_TCSModSwtSts_write/1
  Ground_96/1 -> TCSModSwtSts1_TCSModSwtSts1_write/1
  Ground_97/1 -> TCSnsrTSts_TCSnsrTSts_write/1
  Ground_98/1 -> TCSnsrTSts1_TCSnsrTSts1_write/1
  Ground_99/1 -> TCSRadrTreq_TCSRadrTreq_write/1
  Ground_100/1 -> TCSRadrTreq1_TCSRadrTreq1_write/1
  Ground_101/1 -> TCSRadrTSts_TCSRadrTSts_write/1
  Ground_102/1 -> TCSRadrTSts1_TCSRadrTSts1_write/1
  Ground_103/1 -> TCSTmrOverSts_TCSTmrOverSts_write/1
  Ground_104/1 -> TCSTmrOverSts1_TCSTmrOverSts1_write/1
  Ground_105/1 -> TCSTmrStrtSts_TCSTmrStrtSts_write/1
  Ground_106/1 -> TCSTmrStrtSts1_TCSTmrStrtSts1_write/1
  Ground_107/1 -> TCSTNowSts_TCSTNowSts_write/1
  Ground_108/1 -> TCSTNowSts1_TCSTNowSts1_write/1
  Ground_109/1 -> TgtHvacAirTForMaxTZone_TgtHvacAirTForMaxTZone_write/1
  Ground_110/1 -> ThermoTSteDeoSts1_ThermoTSteDeoSts1_write/1
  Ground_111/1 -> ThrdRowLeSeatHeatgPwrAllwd_ThrdRowLeSeatHeatgPwrAllwd_write/1
  Ground_112/1 -> ThrdRowLeSeatVentnPwrAllwd_ThrdRowLeSeatVentnPwrAllwd_write/1
  Ground_113/1 -> ThrdRowRiSeatHeatgPwrAllwd_ThrdRowRiSeatHeatgPwrAllwd_write/1
  Ground_114/1 -> ThrdRowRiSeatVentnPwrAllwd_ThrdRowRiSeatVentnPwrAllwd_write/1
  Ground_115/1 -> TiOfDeactvnForClimaCtrl_TiOfDeactvnForClimaCtrl_write/1
  Ground_116/1 -> VMMChngNdMtn_VMMChngNdMtn_write/1
  Ground_117/1(Out1) -> AirTFbFromClima_AirTFbFromClima_write/1
  Ground_118/1(Out1) -> AmbTEstimd_AmbTEstimd_write/1
  Ground_119/1 -> CarTiIntForClima_CarTiIntForClima_write/1
  Ground_120/1(Out1) -> CcmCaliSig1_CcmCaliSig1_write/1
  Ground_121/1(Out1) -> CcmCaliSig10_CcmCaliSig10_write/1
  Ground_122/1(Out1) -> CcmCaliSig11_CcmCaliSig11_write/1
  Ground_123/1(Out1) -> CcmCaliSig12_CcmCaliSig12_write/1
  Ground_124/1(Out1) -> CcmCaliSig2_CcmCaliSig2_write/1
  Ground_125/1(Out1) -> CcmCaliSig3_CcmCaliSig3_write/1
  Ground_126/1(Out1) -> CcmCaliSig4_CcmCaliSig4_write/1
  Ground_127/1(Out1) -> CcmCaliSig5_CcmCaliSig5_write/1
  Ground_128/1(Out1) -> CcmCaliSig6_CcmCaliSig6_write/1
  Ground_129/1(Out1) -> CcmCaliSig7_CcmCaliSig7_write/1
  Ground_130/1(Out1) -> CcmCaliSig8_CcmCaliSig8_write/1
  Ground_131/1(Out1) -> CcmCaliSig9_CcmCaliSig9_write/1
  Ground_132/1 -> ClimaActv_ClimaActv_write/1
  Ground_133/1 -> ClimaActvAlrmSts_ClimaActvAlrmSts_write/1
  Ground_134/1 -> ClimaCmptSts_ClimaCmptSts_write/1
  Ground_135/1 -> ClimaDefrstSts_ClimaDefrstSts_write/1
  Ground_136/1 -> ClimaOffReq_ClimaOffReq_write/1
  Ground_137/1 -> ClimaPwrCns_ClimaPwrCns_write/1
  Ground_138/1 -> ClimaPwrCritDes_ClimaPwrCritDes_write/1
  Ground_139/1 -> ClimaPwrNormDes_ClimaPwrNormDes_write/1
  Ground_140/1 -> ClimaTiOfEnd_ClimaTiOfEnd_write/1
  Ground_141/1 -> CmptModForHp_CmptModForHp_write/1
  Ground_142/1 -> CmptmtAirTEstimdAtRowFirstLoResl_CmptmtAirTEstimdAtRowFirstLoResl_write/1
  Ground_143/1(Out1) -> CmptmtAirTEstimdExtd_CmptmtAirTEstimdExtd_write/1
  Ground_144/1 -> CupHoldrActvAllwd_CupHoldrActvAllwd_write/1
  Ground_145/1 -> DefrstMaxSts_DefrstMaxSts_write/1
  Ground_146/1 -> DrvrSeatHeatgPwrAllwd_DrvrSeatHeatgPwrAllwd_write/1
  Ground_147/1 -> DrvrSeatVentnPwrAllwd_DrvrSeatVentnPwrAllwd_write/1
  Ground_148/1 -> EcoClimaSts_EcoClimaSts_write/1
  Ground_149/1 -> EgyDesForClima_EgyDesForClima_write/1
  Ground_150/1(Out1) -> ElecDefrstReq_ElecDefrstReq_write/1
  Ground_151/1 -> EngRunngReqByClima_EngRunngReqByClima_write/1
  Ground_152/1 -> EngSpdIncIdleForClima_EngSpdIncIdleForClima_write/1
  Ground_153/1(Out1) -> EvaprTFrnt_EvaprTFrnt_write/1
  Ground_154/1 -> ExtVentToastFrmClima_ExtVentToastFrmClima_write/1
  Ground_155/1 -> HeatrAirTReq_HeatrAirTReq_write/1
  Ground_156/1 -> HvacAirMFlowEstimd_HvacAirMFlowEstimd_write/1
  Ground_157/1 -> HvacAirMFlowReEstimd_HvacAirMFlowReEstimd_write/1
  Ground_158/1 -> HvacAirMFlowVent_HvacAirMFlowVent_write/1
  Ground_159/1(Out1) -> HvacAirTForRowFirstAtFlrLe_HvacAirTForRowFirstAtFlrLe_write/1
  Ground_160/1(Out1) -> HvacAirTForRowFirstAtFlrRi_HvacAirTForRowFirstAtFlrRi_write/1
  Ground_161/1(Out1) -> HvacAirTForRowFirstAtVentLe_HvacAirTForRowFirstAtVentLe_write/1
  Ground_162/1(Out1) -> HvacAirTForRowFirstAtVentRi_HvacAirTForRowFirstAtVentRi_write/1
  Ground_163/1(Out1) -> HvacAirTForRowSecAtFlrLe_HvacAirTForRowSecAtFlrLe_write/1
  Ground_164/1(Out1) -> HvacAirTForRowSecAtFlrRi_HvacAirTForRowSecAtFlrRi_write/1
  Ground_165/1(Out1) -> HvacAirTForRowSecAtVentLe_HvacAirTForRowSecAtVentLe_write/1
  Ground_166/1(Out1) -> HvacAirTForRowSecAtVentRi_HvacAirTForRowSecAtVentRi_write/1
  Ground_167/1(Out1) -> HvacCondsorTsp_HvacCondsorTsp_write/1
  Ground_168/1 -> HvacCoolgEnaFrnt_HvacCoolgEnaFrnt_write/1
  Ground_169/1 -> HvacCoolgEnaRe_HvacCoolgEnaRe_write/1
  Ground_170/1 -> HvacEvaprTSpFrnt_HvacEvaprTSpFrnt_write/1
  Ground_171/1 -> HvacHeatElmAirMFlowEstimd_HvacHeatElmAirMFlowEstimd_write/1
  Ground_172/1 -> HvacHeatgReq_HvacHeatgReq_write/1
  Ground_173/1(Out1) -> HvacHeatrInletTempReq_HvacHeatrInletTempReq_write/1
  Ground_174/1 -> HvacRecircAct_HvacRecircAct_write/1
  Ground_175/1 -> HvEgyDesForClima_HvEgyDesForClima_write/1
  Ground_176/1 -> InPm25SnsrActnReq_InPm25SnsrActnReq_write/1
  Ground_177/1 -> IntPm25HiPopUp_IntPm25HiPopUp_write/1
  Ground_178/1 -> LvlOfClimaCmft_LvlOfClimaCmft_write/1
  Ground_179/1 -> PassSeatHeatgPwrAllwd_PassSeatHeatgPwrAllwd_write/1
  Ground_180/1 -> PassSeatVentnPwrAllwd_PassSeatVentnPwrAllwd_write/1
  Ground_181/1 -> PostDrvgClimaAvl_PostDrvgClimaAvl_write/1
  Ground_182/1 -> PwrAllwdForElecAirVentn_PwrAllwdForElecAirVentn_write/1
  Ground_183/1 -> PwrEstimdAtStbForClima_PwrEstimdAtStbForClima_write/1
  Ground_184/1 -> RlyCmftForClimaReq_RlyCmftForClimaReq_write/1
  Ground_185/1 -> RlyCrashForClimaReq_RlyCrashForClimaReq_write/1
  Ground_186/1 -> SecRowLeSeatHeatgPwrAllwd_SecRowLeSeatHeatgPwrAllwd_write/1
  Ground_187/1 -> SecRowLeSeatVentnPwrAllwd_SecRowLeSeatVentnPwrAllwd_write/1
  Ground_188/1 -> SecRowRiSeatHeatgPwrAllwd_SecRowRiSeatHeatgPwrAllwd_write/1
  Ground_189/1 -> SecRowRiSeatVentnPwrAllwd_SecRowRiSeatVentnPwrAllwd_write/1
  Ground_190/1 -> SteerWhlHeatgDurgClima_SteerWhlHeatgDurgClima_write/1
  Ground_191/1 -> TgtHvacAirTForRowFirstLe_TgtHvacAirTForRowFirstLe_write/1
  Ground_192/1 -> TgtHvacAirTForRowFirstRi_TgtHvacAirTForRowFirstRi_write/1
  Ground_193/1 -> TgtHvacAirTForRowSecLe_TgtHvacAirTForRowSecLe_write/1
  Ground_194/1 -> TgtHvacAirTForRowSecRi_TgtHvacAirTForRowSecRi_write/1
  Ground_195/1 -> UBoostReqByClima_UBoostReqByClima_write/1
  Ground_196/1(Out1) -> VehMktForPmSnsr_VehMktForPmSnsr_write/1
  Ground_197/1 -> WindIceDetdFrnt_WindIceDetdFrnt_write/1
  Ground_198/1 -> WindIceDetdRe_WindIceDetdRe_write/1
